simulink model "WheelLoads"  (R2020b)
settings: Solver=ode23  StartTime=0.0  StopTime=5  RelTol=0.01  AbsTol=0.01
PreLoadFcn:
  | addpath('Functions');
  | addpath('Functions/geometric');
  | addpath('Parameters');
  | vhclParams;
  | 
  | ELLIPSE = Simulink.Variant('variantMode == 1');
  | CONSTANT_ACC = Simulink.Variant('variantMode == 2');
  | CONSTANT_OUT = Simulink.Variant('variantMode == 3');
  | EXTERNAL_DATA_LOAD = Simulink.Variant('variantMode == 4');
  | EXTERNAL_DATA_ACC = Simulink.Variant('variantMode == 5');
  | if ~exist('F','var')
  |  F = 0;
  | end
  | if ~exist('M','var')
  |  M = 0;
  | end
  | if ~exist('St','var')
  |  St = 0;
  | end
  | if ~exist('Ax','var')
  |  Ax = 0;
  | end
  | if ~exist('Ay','var')
  |  Ay = 0;
  | end
  | if ~exist('StAcc','var')
  |  StAcc = 0;
  | end
InitFcn:
  | [folder, ~, ~] = fileparts(which (bdroot));
  | cd(folder);
undefined symbols (not defined in the file): ARB_bcrnk_frontL, ARB_bcrnk_frontR, ARB_bcrnk_rearL, ARB_bcrnk_rearR, ARB_center_front, ARB_center_rear, ARB_damping_front, ARB_damping_rear, ARB_length_front, ARB_length_rear, ARB_pinSlot_correctionFactor_front, ARB_pinSlot_correctionFactor_rear, ARB_stiffness_front, ARB_stiffness_rear, ARB_TieRod_length_front, ARB_TieRod_length_rear, COG, Damper_coefficient_front, Damper_coefficient_rear, Driver_COG, Driver_mass, DriveTrain_ratio, FS_camber, FS_toe, gravity, Mass, Rim_mass, RodDiameter, RodThickness, RS_camber, RS_toe, sep_lower_front, sep_lower_rear, sep_upper_front, sep_upper_rear, Spring_preload_front, Spring_preload_rear, Spring_stiffness_front, Spring_stiffness_rear, Sprung_mass, Sprung_mass_COG, sym_lower_front, sym_lower_rear, sym_upper_front, sym_upper_rear, Tire_mass, Tire_radius, Unsprung_mass_COG_front, Unsprung_mass_COG_rear, Unsprung_mass_front, Unsprung_mass_rear, util_rodCrossSection, Wheel_inertia, X, X_F, X_R, Y, Z
libraries: sm_lib x400, nesl_utility x70, fl_lib x1
(part 1 of 18)
settings Model: Creator=Adri  LastModifiedBy=Adri
settings SolverCC: ZeroCrossControl=DisableAll
settings DiagnosticsConfigSet: SimMechanicsUnconnectedFramePorts=none
settings ExplorerConfigSet: InternalSimMechanicsExplorerSettings="<ExplorerConfiguration><canonicalview>default</canonicalview><AnimationSetting><BasePlaybackSpeed>1.0</BasePlaybackSpeed><PlaybackSpeedFactor>1.0</PlaybackSpeedFactor></AnimationSetting><TilePanel><TileInfo><Tiles Columns=\"2\" Count=\"4\" Rows=\"2\"><Column Weight=\"0.4998\"/><Column Weight=\"0.5002\"/><Row Weight=\"0.4996\"/><Row Weight=\"0.5004\"/><Tile Height=\"1\" Width=\"1\" X=\"0\" Y=\"0\"/><Tile Height=\"1\" Width=\"1\" X=\"0\" Y=\"1\"/><Tile Height=\"1\" Width=\"1\" X=\"1\" Y=\"0\"/><Tile Height=\"1\" Width=\"1\" X=\"1\" Y=\"1\"/></Tiles></TileInfo><SceneInfo><SceneViewer><BGColor><R>0.60000002384185791</R><G>0.60000002384185791</G><B>0.60000002384185791</B></BGColor><Camera><DoPerspective>on</DoPerspective><Position><X>1.45029999999999992</X><Y>0</Y><Z>0.990999999999999992</Z></Position><Center><X>0.950299999999999923</X><Y>0</Y><Z>0.391000000000000014</Z></Center><Up><X>0</X><Y>0</Y><Z>1</Z></Up><ViewLimDist>1</ViewLimDist><YLim>0.466307658154998583</YLim><XLim>0.836981472268669924</XLim></Camera></SceneViewer><SceneViewer><BGColor><R>0.60000002384185791</R><G>0.60000002384185791</G><B>0.60000002384185791</B></BGColor><Camera><DoPerspective>on</DoPerspective><Position><X>3</X><Y>-3</Y><Z>3</Z></Position><Center><X>0</X><Y>0</Y><Z>0</Z></Center><Up><X>0</X><Y>0</Y><Z>1</Z></Up><ViewLimDist>1</ViewLimDist><YLim>0.466307658154998583</YLim><XLim>0.834328599139450677</XLim></Camera></SceneViewer><SceneViewer><BGColor><R>0.60000002384185791</R><G>0.60000002384185791</G><B>0.60000002384185791</B></BGColor><Camera><DoPerspective>on</DoPerspective><Position><X>-1.09800000000000009</X><Y>0</Y><Z>0.89700000000000002</Z></Position><Center><X>-0.597999999999999976</X><Y>0</Y><Z>0.296999999999999986</Z></Center><Up><X>0</X><Y>0</Y><Z>1</Z></Up><ViewLimDist>1</ViewLimDist><YLim>0.466307658154998583</YLim><XLim>0.837722819896897342</XLim></Camera></SceneViewer><SceneViewer><BGColor><R>0.60000002384185791</R><G>0.60000002384185791</G><B>0.60000002384185791</B></BGColor><Camera><DoPerspective>on</DoPerspective><Position><X>0.299999999999999989</X><Y>3</Y><Z>0.699999999999999956</Z></Position><Center><X>0.299999999999999989</X><Y>0</Y><Z>0.5</Z></Center><Up><X>0</X><Y>0</Y><Z>1</Z></Up><ViewLimDist>1</ViewLimDist><YLim>0.466307658154998583</YLim><XLim>0.835067597012913443</XLim></Camera></SceneViewer></SceneInfo></TilePanel><MechEditorPanel><Cameras><Camera><Id>Camera1</Id><Value><Name>Front suspension</Name><NativeCamera><PositionTrackingFeature>Vehicle.Cameras.Front_suspension_pos.F</PositionTrackingFeature><CenterTrackingFeature>Vehicle.Cameras.Front_suspension_aim.F</CenterTrackingFeature><UpVectorTrackingFeature>World_Frame.W</UpVectorTrackingFeature><CenterTrackingOrigin>on</CenterTrackingOrigin><UpVectorTrackingDirection>+Z</UpVectorTrackingDirection></NativeCamera></Value></Camera><Camera><Id>Camera2</Id><Value><Name>Rear Suspension</Name><NativeCamera><PositionTrackingFeature>Vehicle.Cameras.Rear_suspension_pos.F</PositionTrackingFeature><CenterTrackingFeature>Vehicle.Cameras.Rear_suspension_aim.F</CenterTrackingFeature><UpVectorTrackingFeature>World_Frame.W</UpVectorTrackingFeature><CenterTrackingOrigin>on</CenterTrackingOrigin><UpVectorTrackingDirection>+Z</UpVectorTrackingDirection></NativeCamera></Value></Camera><Camera><Id>Camera3</Id><Value><Name>Vehicle isometric</Name><NativeCamera><PositionTrackingFeature>Vehicle.Cameras.Vehicle_Isometric_pos.F</PositionTrackingFeature><CenterTrackingFeature>Vehicle.Cameras.Chassis_Ref</CenterTrackingFeature><UpVectorTrackingFeature>World_Frame.W</UpVectorTrackingFeature><CenterTrackingOrigin>on</CenterTrackingOrigin><UpVectorTrackingDirection>+Z</UpVectorTrackingDirection></NativeCamera></Value></Camera><Camera><Id>Camera8</Id><Value><Name>Top</Name><NativeCamera><PositionTrackingFeature>Vehicle.Cameras.Top_pos.F</PositionTrackingFeature><CenterTrackingFeature>Vehicle.Cameras.Chassis_Ref</CenterTrackingFeature><UpVectorTrackingFeature>World_Frame.W</UpVectorTrackingFeature><CenterTrackingOrigin>on</CenterTrackingOrigin><UpVectorTrackingDirection>+X</UpVectorTrackingDirection></NativeCamera></Value></Camera><Camera><Id>Camera9</Id><Value><Name>Front</Name><NativeCamera><PositionTrackingFeature>Vehicle.Cameras.Front.F</PositionTrackingFeature><CenterTrackingFeature>Vehicle.Cameras.Chassis_Ref</CenterTrackingFeature><UpVectorTrackingFeature>Vehicle.Cameras.Chassis_Ref</UpVectorTrackingFeature><CenterTrackingOrigin>on</CenterTrackingOrigin><UpVectorTrackingDirection>+Z</UpVectorTrackingDirection></NativeCamera></Value></Camera><Camera><Id>Camera10</Id><Value><Name>Left</Name><NativeCamera><PositionTrackingFeature>Vehicle.Cameras.Left.F</PositionTrackingFeature><CenterTrackingFeature>Vehicle.Cameras.Left_aim.F</CenterTrackingFeature><UpVectorTrackingFeature>Vehicle.Cameras.Chassis_Ref</UpVectorTrackingFeature><CenterTrackingOrigin>on</CenterTrackingOrigin><UpVectorTrackingDirection>+Z</UpVectorTrackingDirection></NativeCamera></Value></Camera><Camera><Id>Camera11</Id><Value><Name>Right</Name><NativeCamera><PositionTrackingFeature>Vehicle.Cameras.Right.F</PositionTrackingFeature><CenterTrackingFeature>Vehicle.Cameras.Right_aim.F</CenterTrackingFeature><UpVectorTrackingFeature>Vehicle.Cameras.Chassis_Ref</UpVectorTrackingFeature><CenterTrackingOrigin>on</CenterTrackingOrigin><UpVectorTrackingDirection>+Z</UpVectorTrackingDirection></NativeCamera></Value></Camera><Camera><Id>Camera12</Id><Value><Name>Rear</Name><NativeCamera><PositionTrackingFeature>Vehicle.Cameras.Rear.F</PositionTrackingFeature><CenterTrackingFeature>Vehicle.Cameras.Chassis_Ref</CenterTrackingFeature><UpVectorTrackingFeature>Vehicle.Cameras.Chassis_Ref</UpVectorTrackingFeature><CenterTrackingOrigin>on</CenterTrackingOrigin><UpVectorTrackingDirection>+Z</UpVectorTrackingDirection></NativeCamera></Value></Camera></Cameras><GfxPanelCameraMap><MapElement CameraMLId=\"Camera1\" GfxPanelIndex=\"0\"/><MapElement CameraMLId=\"Camera3\" GfxPanelIndex=\"1\"/><MapElement CameraMLId=\"Camera2\" GfxPanelIndex=\"2\"/><MapElement CameraMLId=\"Camera10\" GfxPanelIndex=\"3\"/></GfxPanelCameraMap><VideoCreator><PlaybackSpeedRatio>1.0</PlaybackSpeedRatio><FrameRate>30</FrameRate><VideoFormat>Motion JPEG AVI</VideoFormat><FrameSize>auto</FrameSize></VideoCreator></MechEditorPanel></ExplorerConfiguration>"

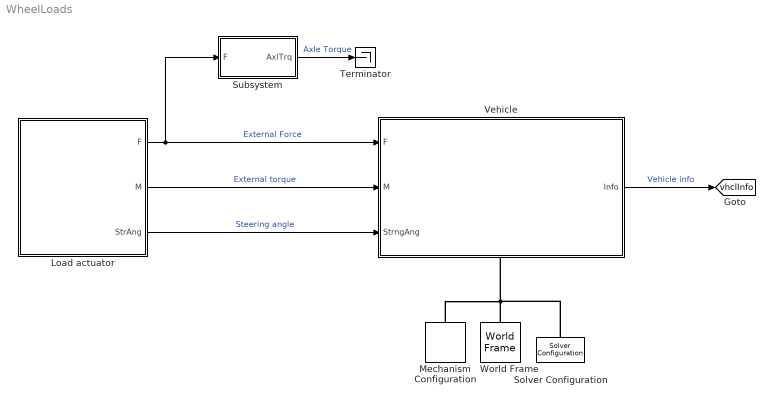
== system WheelLoads ==
  Load actuator : Subsystem  Variant=on  mask{time=5, mode=Acceleration ellipse, e_axBrk=2, e_axAcc=2, e_ay=2.1, e_st=120, c_ax=1.63, c_ay=0, c_st=0, c_frcMode=Step, FL_Fx=0, FL_Fy=0, FL_Fz=750, FR_Fx=0, FR_Fy=0, FR_Fz=750, RL_Fx=0, RL_Fy=0, RL_Fz=800, RR_Fx=0, RR_Fy=0, RR_Fz=800, FL_Mx=0, FL_My=0, FL_Mz=0, FR_Mx=0, FR_My=0, FR_Mz=0, RL_Mx=0, RL_My=0, RL_Mz=0, RR_Mx=0, RR_My=0, RR_Mz=0, m_st=0, extMode=Loads, extAx=Ax, extAy=Ay, extStAcc=St, extF=F, extM=M, extSt=St, mass=Mass, wBias=(COG(1)-X_R(25))/(X_F(25)-X_R(25)), cog=(COG(3))*1e-3, wheelbase=(X_F(25)-X_R(25))*1e-3, track=X_F(26)*2*1e-3, tireR=Tire_radius*1e-3, driveRatio=DriveTrain_ratio, F=F, M=M, St=0, timeStep=0.01}
      mask prompts: e_axBrk "Ax - brake"; e_axAcc "Ax - acceleration"; e_ay "Ay"; e_st "Max steering"; c_ax "Ax"; c_ay "Ay"; c_st "Steering"; c_frcMode "Force application"; FL_Fx "Fx"; FL_Fy "Fy"; FL_Fz "Fz"; FR_Fx "Fx"; FR_Fy "Fy"; FR_Fz "Fz"; RL_Fx "Fx"; RL_Fy "Fy"; RL_Fz "Fz"; RR_Fx "Fx"; RR_Fy "Fy"; RR_Fz "Fz"; FL_Mx "Mx"; FL_My "My"; FL_Mz "Mz"; FR_Mx "Mx"; FR_My "My"; FR_Mz "Mz"; RL_Mx "Mx"; RL_My "My"; RL_Mz "Mz"; RR_Mx "Mx"; RR_My "My"; RR_Mz "Mz"; m_st "Steering"; extAx "Ax"; extAy "Ay"; extStAcc "Steering"; extF "Force"; extM "Moment"; extSt "Steering"; wBias "Weight distribution"; cog "COG height"; tireR "Tire Radius"; driveRatio "Drivetrain ratio"; F "Force"; M "Moment"; St "Steering"; timeStep "Time step"
      mask init:
      | p = Simulink.Mask.get(gcb);
      | switch get_param(gcb,'mode')
      |     case 'Acceleration ellipse'
      |         assignin('base','variantMode',1);
      |     case 'Constant acceleration'
      |         assignin('base','variantMode',2);
      |     case 'Manual'
      |         assignin('base','variantMode',3);
      |     case 'External data'
      |         if strcmp(get_param(gcb,'extMode'),'Loads')
      |             assignin('base','variantMode',4);
      |         elseif strcmp(get_param(gcb,'extMode'),'Acceleration')
      |             assignin('base','variantMode',5);
      |         end
      | end
      | set_param(gcs, 'StopTime', num2str(time))
      mask TypeOptions mode: Acceleration ellipse | Constant acceleration | Manual | External data
      mask Callback mode:
      | p = Simulink.Mask.get(gcb);
      | switch get_param(gcb,'mode')
      |     case 'Acceleration ellipse'
      |         assignin('base','variantMode',1);
      |         p.getDialogControl('ellipseDef_Container').Visible='on';
      |         p.getDialogControl('accVal_Container').Visible='off';
      |         p.getDialogControl('manual_Container').Visible='off';
      |         p.getDialogControl('external_Container').Visible='off';
      |         p.getDialogControl('vhclParams_Container').Visible='on';
      |     case 'Constant acceleration'
      |         assignin('base','variantMode',2);
      |         p.getDialogControl('ellipseDef_Container').Visible='off';
      |         p.getDialogControl('accVal_Container').Visible='on';
      |         p.getDialogControl('manual_Container').Visible='off';
      |         p.getDialogControl('external_Container').Visible='off';
      |         p.getDialogControl('vhclParams_Container').Visible='on';
      |     case 'Manual'
      |         assignin('base','variantMode',3);
      |         p.getDialogControl('ellipseDef_Container').Visible='off';
      |         p.getDialogControl('accVal_Container').Visible='off';
      |         p.getDialogControl('manual_Container').Visible='on';
      |         p.getDialogControl('external_Container').Visible='off';
      |         p.getDialogControl('vhclParams_Container').Visible='off';
      |     case 'External data'
      |         assignin('base','variantMode',4);
      |         p.getDialogControl('ellipseDef_Container').Visible='off';
      |         p.getDialogControl('accVal_Container').Visible='off';
      |         p.getDialogControl('manual_Container').Visible='off';
      |         p.getDialogControl('external_Container').Visible='on';
      |         p.getDialogControl('vhclParams_Container').Visible='off';
      | end
      mask TypeOptions c_frcMode: Step | Ramp
      mask TypeOptions extMode: Loads | Acceleration
      mask Callback extMode:
      | p = Simulink.Mask.get(gcb);
      | switch get_param(gcb,'extMode')
      |     case 'Loads'
      |         assignin('base','variantMode',4);
      |         p.getDialogControl('extLoad_Container').Visible='on';
      |         p.getDialogControl('extAcc_Container').Visible='off';
      |     case 'Acceleration'
      |         assignin('base','variantMode',5);
      |          p.getDialogControl('extLoad_Container').Visible='off';
      |         p.getDialogControl('extAcc_Container').Visible='on';
      | end
      mask Callback extAx:
      | try
      |     if strcmp(get_param(gcb,'mode'),'External data') && strcmp(get_param(gcb,'extMode'),'Acceleration')
      |         t = evalin('base',[get_param(gcb,'extAx') '.time(end)']);
      |         set_param('WheelLoads_optim', 'StopTime', num2str(t));
      |         set_param(gcb,'time',num2str(t));
      |     end
      | catch err
      |     disp(err)
      | end
      mask Callback extAy:
      | try
      |     if strcmp(get_param(gcb,'mode'),'External data') && strcmp(get_param(gcb,'extMode'),'Acceleration')
      |         t = evalin('base',[get_param(gcb,'extAy') '.time(end)']);
      |         set_param('WheelLoads_optim', 'StopTime', num2str(t));
      |         set_param(gcb,'time',num2str(t));
      |     end
      | catch err
      |     disp(err)
      | end
      mask Callback extStAcc:
      | try
      |     if strcmp(get_param(gcb,'mode'),'External data') && strcmp(get_param(gcb,'extMode'),'Acceleration')
      |         t = evalin('base',[get_param(gcb,'extStAcc') '.time(end)']);
      |         set_param('WheelLoads_optim', 'StopTime', num2str(t));
      |         set_param(gcb,'time',num2str(t));
      |     end
      | catch err
      |     disp(err)
      | end
      mask Callback extF:
      | try
      |     if strcmp(get_param(gcb,'mode'),'External data') && strcmp(get_param(gcb,'extMode'),'Loads')
      |         t = evalin('base',[get_param(gcb,'extF') '.Fx.time(end)']);
      |         set_param('WheelLoads_optim', 'StopTime', num2str(t));
      |         set_param(gcb,'time',num2str(t));
      |     end
      | catch err
      |     disp(err)
      | end
      mask Callback extM:
      | try
      |     if strcmp(get_param(gcb,'mode'),'External data') && strcmp(get_param(gcb,'extMode'),'Loads')
      |         t = evalin('base',[get_param(gcb,'extM') '.Mx.time(end)']);
      |         set_param('WheelLoads_optim', 'StopTime', num2str(t));
      |         set_param(gcb,'time',num2str(t));
      |     end
      | catch err
      |     disp(err)
      | end
      mask Callback extSt:
      | try
      |     if strcmp(get_param(gcb,'mode'),'External data') && strcmp(get_param(gcb,'extMode'),'Loads')
      |         t = evalin('base',[get_param(gcb,'extSt') '.time(end)']);
      |         set_param('WheelLoads_optim', 'StopTime', num2str(t));
      |         set_param(gcb,'time',num2str(t));
      |     end
      | catch err
      |     disp(err)
      | end
      mask DialogControl DescGroupVar Prompt: Load actuator
      mask DialogControl DescTextVar Prompt: Outputs load values for each wheel, which are determined based on the configuration parameters
      mask DialogControl ellipseDef_Container Prompt: Ellipse definition
      mask DialogControl e_axBrk Tooltip: Maximum longitudinal acceleration while braking (g)
      mask DialogControl e_axAcc Tooltip: Maximum longitudinal acceleration while accelerating (g)
      mask DialogControl e_ay Tooltip: Maximum lateral acceleration (g)
      mask DialogControl e_st Tooltip: Maximum steering angle (deg)
      mask DialogControl accVal_Container Prompt: Acceleration values
      mask DialogControl c_ax Tooltip: Longitudinal acceleration. Positive for acceleration and negative for braking (g)
      mask DialogControl c_ay Tooltip: Lateral acceleration. Positive for left turn (negative for right turn). (g)
      mask DialogControl c_st Tooltip: Steering angle (deg)
      mask DialogControl manual_Container Prompt: Load values
      mask DialogControl Container8 Prompt: Force
      mask DialogControl Container7 Prompt: FL
      mask DialogControl Container6 Prompt: FR
      mask DialogControl Container5 Prompt: RL
      mask DialogControl Container10 Prompt: RR
      mask DialogControl Container9 Prompt: Torque
      mask DialogControl Container11 Prompt: FL
      mask DialogControl Container12 Prompt: FR
      mask DialogControl Container13 Prompt: RL
      mask DialogControl Container14 Prompt: RR
      mask DialogControl m_st Tooltip: Steering angle (deg)
      mask DialogControl external_Container Prompt: External data
      mask DialogControl extSt Tooltip: Steering angle (deg)
      mask DialogControl Container20 Prompt: Vehicle Parameters
      mask DialogControl mass Tooltip: Vehicle total mass (kg)
      mask DialogControl wBias Tooltip: Fraction of weight in the front axle (e.g. 0.45)
      mask DialogControl cog Tooltip: Center of gravity height (m)
      mask DialogControl wheelbase Tooltip: Wheelbase (m)
      mask DialogControl track Tooltip: Track (m)
      mask DialogControl Container15 Prompt: Internal
      mask Display: image('$imagefile'); % Use "Edit Image" on toolstrip to change.
  Mechanism Configuration : sm_lib/Utilities/Mechanism Configuration  UniformGravity=Constant  GravityVector=[0 0 -gravity]  GravityVectorUnits=m/s^2  GravityUnits=m/s^2  LinearizationDelta=0.001  JointModeTransitionNonlinearIterations=2
  Solver Configuration : nesl_utility/Solver Configuration  PhysicalDomain=network_engine_domain  SubClassName=solver  LeftPortType=input  RightPortType=generic  Tones=[]  Harmonics=[]  SimulateNoise=off  Profile=off  UseLocalSampling=off  EnableSwitchedLinearOptims=on  FrequencyDomain=off  UseCCode=off  RelTol=0.001  AbsTol=1e-06  MinStep=1e-09  ToWorkspace=off  RevertToSquareIcSolve=off  NumHomotopyIterations=100  PhasorMode=off  PhasorModeNumHarmonics=1  DoDC=off  ResidualTolerance=1e-09  UseLocalSolver=off  LocalSolverChoice=NE_BACKWARD_EULER_ADVANCER  LocalSolverSampleTime=0.001  DoFixedCost=off  MaxNonlinIter=3  MaxModeIter=2  ComputeImpulses=off  ImpulseIterations=2  LinearAlgebra=Sparse  EquationFormulation=NE_TIME_EF  DelaysMemoryBudget=1024  AutomaticFiltering=on  FilteringTimeConstant=0.1  PartitionStorageMethod=AS_NEEDED  PartitionMemoryBudget=1024  PartitionMethod=FAST
  Subsystem : Subsystem
  Terminator : Terminator
  Vehicle : Subsystem
      settings: PortSchema="{\"entries\":[{\"content\":{\"sides\":[{\"content\":{\"connectorIds\":[\"In1\",\"In2\",\"In3\"]},\"type\":\"ConnectorPlacement.EquallySpacedRectSide\",\"uuid\":\"4ba0f89d-2af1-4655-8f71-325e5ad02245\"},{\"content\":{\"connectorIds\":[\"Out1\"],\"side\":\"RIGHT\"},\"type\":\"ConnectorPlacement.EquallySpacedRectSide\",\"uuid\":\"e40e4122-155c-4528-902a-77dc8216dbbe\"},{\"content\":{\"connectorIds\":[],\"side\":\"TOP\"},\"type\":\"ConnectorPlacement.EquallySpacedRectSide\",\"uuid\":\"80d51cd1-2f42-4c41-ac4a-812297993a3c\"},{\"content\":{\"connectorIds\":[\"RConn1\"],\"side\":\"BOTTOM\"},\"type\":\"ConnectorPlacement.EquallySpacedRectSide\",\"uuid\":\"0457f4ce-e2d2-401b-a73a-336035eb9210\"}]},\"type\":\"ConnectorPlacement.EquallySpacedConnector\",\"uuid\":\"a9bd6a3f-05ff-481c-9682-f76d449faaea\"}],\"packageUris\":[\"http://schema.mathworks.com/mf0/Connector/R2020b\"],\"version\":\"1.0\"}"
  World Frame : sm_lib/Frames and Transforms/World Frame
  tag scope: vhclInfo=global
  wires:
  Vehicle/1(Info) -> [vhclInfo]  "Vehicle info"
  Load actuator/3(StrAng) -> Vehicle/3(StrngAng)  "Steering angle"
  Load actuator/1(F) -> Subsystem/1(F), Vehicle/1(F)  "External Force"
  Load actuator/2(M) -> Vehicle/2(M)  "External torque"
  Subsystem/1(AxlTrq) -> Terminator/1  "Axle Torque"
  connections:
  World Frame/RConn1 -- Mechanism Configuration/RConn1 -- Solver Configuration/RConn1 -- Vehicle/RConn1

== system WheelLoads/Load actuator ==
  note: 1) Add Subsystem or Model blocks as valid variant choices.
      | 2) You cannot connect blocks at this level. At simulation, connectivity is automatically
      | determined, based on the active variant and port name matching.
  Constant acc : Subsystem  VariantControl=CONSTANT_ACC  mask{Fx=0, Fy=0, Fz=0, mass=mass, wBias=wBias, cog=cog, wheelbase=wheelbase, track=track, tireR=tireR, driveRatio=driveRatio, c_ax=c_ax, c_ay=c_ay, frcMode=c_frcMode, time=time, timeStep=timeStep, St=0, c_st=c_st}
      mask prompts: tireR "Tire Radius"; driveRatio "Drivetrain Ratio"; c_ax "Ax Brake"; c_ay "Ay"; frcMode "Force Mode"; timeStep "time step"
      mask init:
      | [Fx, Fy, Fz, St, Ax, Ay] = constAcc_init(gcb);
  Constant output : Subsystem  VariantControl=CONSTANT_OUT
  Ellipse : Subsystem  VariantControl=ELLIPSE  mask{Ax=0, Ay=0, Fx=0, Fy=0, Fz=0, St=0, mass=mass, wBias=wBias, cog=cog, wheelbase=wheelbase, track=track, tireR=tireR, driveRatio=driveRatio, e_axBrk=e_axBrk, e_axAcc=e_axAcc, e_ay=e_ay, e_st=e_st, time=time, timeStep=timeStep}
      mask prompts: tireR "Tire Radius"; driveRatio "Drivetrain Ratio"; e_axBrk "Ax Brake"; e_axAcc "Ax Acceleration"; e_ay "Ay"; e_st "Steering"; timeStep "time step"
      mask init:
      | [Fx, Fy, Fz, St, Ax, Ay] = ellipseLoad_init(gcb);
  External data - acc : Subsystem  VariantControl=EXTERNAL_DATA_ACC  mask{Fx=0, Fy=0, Fz=0, St=extStAcc, mass=mass, wBias=wBias, cog=cog, wheelbase=wheelbase, track=track, tireR=tireR, driveRatio=driveRatio, ext_ax=extAx, ext_ay=extAy}
      mask prompts: tireR "Tire Radius"; driveRatio "Drivetrain Ratio"; ext_ax "Ax"; ext_ay "Ay"
      mask init:
      | [Fx, Fy, Fz] = extAcc_init(gcb);
  External data - load : Subsystem  VariantControl=EXTERNAL_DATA_LOAD  mask{F=extF, M=extM, St=extSt}
  F : Outport
  M : Outport  Port=2
  StrAng : Outport  Port=3

== system WheelLoads/Subsystem ==
  note: My
  note: Mx
  F : Inport
  Add : Sum
  Constant : Constant  Value=[0 0 0 0]'
  Dead Zone : DeadZone  LowerValue=-inf  UpperValue=0  SaturateOnIntegerOverflow=on
  Divide : Product  Inputs=/*
  Gain : Gain  Gain=-1
  Gain1 : Gain
  Matrix Concatenate1 : Concatenate  NumInputs=3  Mode=Multidimensional array  ConcatenateDimension=2
  Product : Product
  Product1 : Product
  Select all Fx : Selector  NumberOfDimensions=2  InputPortWidth=4  IndexOptions=Select all,Index vector (dialog)  Indices=1,1  OutputSizes=1,1
  Select all Fx1 : Selector  NumberOfDimensions=2  InputPortWidth=4  IndexOptions=Select all,Index vector (dialog)  Indices=1,2  OutputSizes=1,1
  Terminator : Terminator
  Tire radius : Constant  Value=228.6*1e-3
  Transmision ratio : Constant  Value=DriveTrain_ratio
  AxlTrq : Outport
  wires:
  Select all Fx/1 -> Product/2
  Transmision ratio/1 -> Divide/1
  Product/1 -> Gain/1, Divide/2
  Gain/1 -> Matrix Concatenate1/2, Add/2  "AxlTrq - My"
  Divide/1 -> Dead Zone/1
  Dead Zone/1 -> Add/1  "Mounting plate torque"
  Add/1 -> Terminator/1
  Tire radius/1 -> Product1/1, Product/1
  Select all Fx1/1 -> Product1/2
  F/1 -> Select all Fx1/1, Select all Fx/1
  Gain1/1 -> Matrix Concatenate1/1  "AxlTrq - Mx"
  Product1/1 -> Gain1/1
  Matrix Concatenate1/1 -> AxlTrq/1
  Constant/1 -> Matrix Concatenate1/3  "AxlTrq - Mz"
